simulink model "ofdm_txrx_supermimo_coded"  (Simulink 7.7)
settings: Solver=VariableStepDiscrete  SolverMode=SingleTasking  StartTime=0.0  StopTime=2*simOnly_simLength  RelTol=1e-3
PostLoadFcn:
  | ofdm_rx_supermimo_init
InitFcn:
  | ofdm_rx_supermimo_init
StartFcn:
  | ofdm_rx_supermimo_init
values from neighbouring files (symbols this model uses):
  ActionID_AFTransmit = 31    [ofdm_tx_supermimo_init.m]
  ActionID_Disabled = 0    [ofdm_tx_supermimo_init.m]
  ActionID_SetFlagA = 62    [ofdm_tx_supermimo_init.m]
  ActionID_SetFlagB = 61    [ofdm_tx_supermimo_init.m]
  BER_RAM_init_size = 2048    [ofdm_tx_supermimo_init.m]
  channel_h = [1 0; 1 0] * 0.3    [ofdm_tx_supermimo_init.m]
  CPLength = 16    [ofdm_tx_supermimo_init.m]
  longCorr_coef_i = [-4*real(LTS) 0]    [ofdm_rx_supermimo_init.m]
  longCorr_coef_q = [4*imag(LTS) 0]    [ofdm_rx_supermimo_init.m]
  max_CP_length = 16    [ofdm_tx_supermimo_init.m]
  max_num_baseRateSymbols = 31    [ofdm_tx_supermimo_init.m]
  max_num_subcarriers = 64    [ofdm_tx_supermimo_init.m]
  max_num_trainingSymbols = 15    [ofdm_tx_supermimo_init.m]
  max_numBytes = 16384    [ofdm_tx_supermimo_init.m]
  max_OFDM_symbols = 1023    [ofdm_tx_supermimo_init.m]
  modConstellation_qam16 = .75*[1 3 -1 -3]./3    [ofdm_tx_supermimo_init.m]
  modConstellation_qam64 = 0.875*[3 1 5 7 -3 -1 -5 -7]./7    [ofdm_tx_supermimo_init.m]
  modConstellation_qpsk = [1 -1]./sqrt(2)    [ofdm_tx_supermimo_init.m]
  numBaseRateSymbols = numBaseRateSymbols *2    [ofdm_tx_supermimo_init.m]
  numSubcarriers = 64    [ofdm_tx_supermimo_init.m]
  numTrainingSymbols = 2    [ofdm_tx_supermimo_init.m]
  pktTiming_count_payloadStart = pktTiming_count_lsStart + pktTiming_ls_num*pktTiming_ls_length    [ofdm_rx_supermimo_init.m]
  preamble = preamble_scale*[shortsyms_10 longsyms_2]    [ofdm_tx_supermimo_init.m]
  preamble_ROM = preamble_I_ROM + preamble_Q_ROM*2^16    [ofdm_tx_supermimo_init.m]
  RAM_init_size = max_numBytes    (= 16384)    [ofdm_tx_supermimo_init.m]
  RxDataScrambling_Seq = [40 198 78 63 82 173 102 245 48 111 172 115 147 230 216 93 72 65 62 2 205 242 122 90 128 83 105 97 73 10 5 252]    [ofdm_tx_supermimo_init.m]
  shortSymbol_time = shortSymbol_time(1:16).'    [ofdm_tx_supermimo_init.m]
  simOnly_numSamples = length(preamble)+( (numSubcarriers+CPLength)*(numTrainingSymbols + numBaseRateSymbols + numFullRateSymbols/2) )    [ofdm_tx_supermimo_init.m]
  train = [train train]    [ofdm_tx_supermimo_init.m]
  TxDataScrambling_Seq = [40 198 78 63 82 173 102 245 48 111 172 115 147 230 216 93 72 65 62 2 205 242 122 90 128 83 105 97 73 10 5 252]    [ofdm_tx_supermimo_init.m]
undefined symbols (not defined in the file or in its folder): AntA, AntB, BaseRate, Down, gcbh, numBuffs, numBytes, Up, xlBlockLoadCallback, xlGetNormalizedPeriod, xlipgetpartsetting
libraries: xbsIndex_r4 x5430
(part 23 of 49)

== system ofdm_txrx_supermimo_coded/OFDM Rx MIMO/Packet_Detection/RSSI-based PktDet/DIFS Counter ==
  Busy : Inport
  Idle : Inport  Port=2
  Convert1 : xbsIndex_r4/Convert  gui_display_data_type=Fixed-point  arith_type=Unsigned  n_bits=1  bin_pt=0  float_type=Single  exp_bits=8  fraction_bits=24  quantization=Truncate  overflow=Wrap  en=off  latency=0  dbl_ovrd=off  pipeline=off  block_type=convert
      settings: block_version=VER_STRING_GOES_HERE
  Convert2 : xbsIndex_r4/Convert  gui_display_data_type=Fixed-point  arith_type=Boolean  n_bits=1  bin_pt=0  float_type=Single  exp_bits=8  fraction_bits=24  quantization=Truncate  overflow=Wrap  en=off  latency=0  dbl_ovrd=off  pipeline=off  block_type=convert
      settings: block_version=VER_STRING_GOES_HERE
  Convert3 : xbsIndex_r4/Convert  gui_display_data_type=Fixed-point  arith_type=Boolean  n_bits=1  bin_pt=0  float_type=Single  exp_bits=8  fraction_bits=24  quantization=Truncate  overflow=Wrap  en=off  latency=0  dbl_ovrd=off  pipeline=off  block_type=convert
      settings: block_version=VER_STRING_GOES_HERE
  Counter1 : xbsIndex_r4/Counter  cnt_type=Free Running  cnt_to=Inf  operation=Up  start_count=0  cnt_by_val=1  arith_type=Unsigned  n_bits=10  bin_pt=0  load_pin=off  rst=on  en=on  explicit_period=off  period=4  dbl_ovrd=off  use_behavioral_HDL=off  implementation=Fabric  use_rpm=off  block_type=counter
  Inverter1 : xbsIndex_r4/Inverter  en=off  latency=0  dbl_ovrd=off  block_type=inv
      settings: xl_area=[0 0 0 0 0 0 0]  block_version=8.2
  Logical1 : xbsIndex_r4/Logical  logical_function=OR  inputs=2  en=off  latency=0  precision=Full  arith_type=Unsigned  n_bits=16  bin_pt=0  align_bp=on  dbl_ovrd=off  block_type=logical
  Relational3 : xbsIndex_r4/Relational  mode=a>b  en=off  latency=0  dbl_ovrd=off  block_type=relational
      settings: block_version=VER_STRING_GOES_HERE
  S-R Latch : Subsystem
  Idle for DIFS : Outport
  tag scope: regPktDet_idleCounter=global
  wires:
  Convert1/1 -> Idle for DIFS/1
  Idle/1 -> Convert2/1
  S-R Latch/1(Q) -> Convert1/1
  Relational3/1 -> S-R Latch/2(S)
  [regPktDet_DIFSperiod] -> Relational3/2
  Counter1/1 -> Relational3/1, [regPktDet_idleCounter]
  Inverter1/1 -> Logical1/2
  Logical1/1 -> Counter1/1
  Busy/1 -> Convert3/1
  Convert2/1 -> Counter1/2, Inverter1/1
  Convert3/1 -> S-R Latch/1(R), Logical1/1

== system ofdm_txrx_supermimo_coded/OFDM Rx MIMO/Packet_Detection/RSSI-based PktDet/MIMO Logic ==
  Det A : Inport
  Det B : Inport  Port=2
  Convert{1..4,7} (5 alike) : xbsIndex_r4/Convert  gui_display_data_type=Fixed-point  arith_type=Unsigned  n_bits=1  bin_pt=0  float_type=Single  exp_bits=8  fraction_bits=24  quantization=Truncate  overflow=Flag as error  en=off  latency=0  dbl_ovrd=off  pipeline=off  block_type=convert
      settings: block_version=10.1.3
  Logical : xbsIndex_r4/Logical  logical_function=AND  inputs=2  en=off  latency=0  precision=Full  arith_type=Unsigned  n_bits=16  bin_pt=0  align_bp=on  dbl_ovrd=off  block_type=logical
  Logical1 : xbsIndex_r4/Logical  logical_function=AND  inputs=2  en=off  latency=0  precision=Full  arith_type=Unsigned  n_bits=16  bin_pt=0  align_bp=on  dbl_ovrd=off  block_type=logical
  Logical2 : xbsIndex_r4/Logical  logical_function=OR  inputs=2  en=off  latency=0  precision=Full  arith_type=Unsigned  n_bits=16  bin_pt=0  align_bp=on  dbl_ovrd=off  block_type=logical
  Logical3 : xbsIndex_r4/Logical  logical_function=AND  inputs=2  en=off  latency=0  precision=Full  arith_type=Unsigned  n_bits=16  bin_pt=0  align_bp=on  dbl_ovrd=off  block_type=logical
  Mux : xbsIndex_r4/Mux  inputs=2  en=off  latency=0  precision=Full  arith_type=Unsigned  n_bits=16  bin_pt=14  quantization=Truncate  overflow=Wrap  dbl_ovrd=off  block_type=mux
  PktDet : Outport
  wires:
  [regPktDet_pktDetMode] -> Convert4/1
  [regPktDet_pktDetMask_A] -> Convert2/1
  [regPktDet_pktDetMask_B] -> Convert3/1
  Det A/1 -> Convert7/1
  Det B/1 -> Convert1/1
  Convert7/1 -> Logical/1
  Convert2/1 -> Logical/2
  Convert1/1 -> Logical1/1
  Convert3/1 -> Logical1/2
  Logical/1 -> Logical2/1, Logical3/1
  Logical1/1 -> Logical2/2, Logical3/2
  Logical2/1 -> Mux/3
  Convert4/1 -> Mux/1
  Logical3/1 -> Mux/2
  Mux/1 -> PktDet/1

== system ofdm_txrx_supermimo_coded/OFDM Rx MIMO/Packet_Detection/RSSI-based PktDet/Mid-Packet RSSI Register ==
  AntB : Inport
  AntA : Inport  Port=2
  Payload : Inport  Port=3
  Concat1 : xbsIndex_r4/Concat  num_inputs=2  dbl_ovrd=off  block_type=concat
      settings: block_version=10.1.3
  Convert4 : xbsIndex_r4/Convert  gui_display_data_type=Fixed-point  arith_type=Boolean  n_bits=1  bin_pt=0  float_type=Single  exp_bits=8  fraction_bits=24  quantization=Truncate  overflow=Wrap  en=off  latency=0  dbl_ovrd=off  pipeline=off  block_type=convert
      settings: block_version=10.1.3
  Convert5 : xbsIndex_r4/Convert  gui_display_data_type=Fixed-point  arith_type=Unsigned  n_bits=16  bin_pt=0  float_type=Single  exp_bits=8  fraction_bits=24  quantization=Truncate  overflow=Wrap  en=off  latency=0  dbl_ovrd=off  pipeline=off  block_type=convert
      settings: block_version=10.1.3
  Convert6 : xbsIndex_r4/Convert  gui_display_data_type=Fixed-point  arith_type=Unsigned  n_bits=16  bin_pt=0  float_type=Single  exp_bits=8  fraction_bits=24  quantization=Truncate  overflow=Wrap  en=off  latency=0  dbl_ovrd=off  pipeline=off  block_type=convert
      settings: block_version=10.1.3
  Terminator : Terminator
  To Register2 : xbsIndex_r4/To Register  shared_memory_name='midPacketRSSI'  init=0  ownership=Locally owned and initialized  explicit_data_type=on  gui_display_data_type=Fixed-point  arith_type=Unsigned  n_bits=32  bin_pt=0  preci_type=Single  dbl_ovrd=off  block_type=toreg
      settings: AttributesFormatString=<< %<shared_memory_name> >>  infoedit="Register block that writes data to a shared memory register.  Delay of one sample period."  block_version=10.1.3
  posedge1 : Subsystem
  wires:
  Payload/1 -> posedge1/1(In)
  posedge1/1(Out) -> Convert4/1
  Concat1/1 -> To Register2/1
  To Register2/1 -> Terminator/1
  Convert4/1 -> To Register2/2
  Convert5/1 -> Concat1/1
  Convert6/1 -> Concat1/2
  AntB/1 -> Convert5/1
  AntA/1 -> Convert6/1

== system ofdm_txrx_supermimo_coded/OFDM Rx MIMO/Packet_Detection/RSSI-based PktDet/RSSI Clock Generator ==
  note: The RSSI ADC on the radio board can be clocked up to 20MHz.
      | We clock it at 10MHz (1/4 the I/Q samping rate). This
      | clock is generated here by a 20MHz 2-bit counter. If the LBS is used,
      | the RSSI clock will be CLK/2 (10MHz). If the second LSB is used,
      | it will be CLK/4 (5MHz).
  2-bit Counter : xbsIndex_r4/Counter  cnt_type=Free Running  cnt_to=Inf  operation=Up  start_count=0  cnt_by_val=1  arith_type=Unsigned  n_bits=2  bin_pt=0  load_pin=off  rst=off  en=off  explicit_period=on  period=2  dbl_ovrd=off  use_behavioral_HDL=off  implementation=Fabric  use_rpm=off  block_type=counter
      settings: block_version=VER_STRING_GOES_HERE
  LSB : xbsIndex_r4/Slice  nbits=1  boolean_output=off  mode=Lower Bit Location + Width  bit1=0  base1=MSB of Input  bit0=0  base0=LSB of Input  dbl_ovrd=off  block_type=slice
      settings: infoedit="Extracts a given range of bits from each input sample and presents it at the output.  The output type is ordinarily unsigned with binary point at zero, but can be Boolean when the slice is one bit wide.<P><P>Hardware notes: In hardware this block costs nothing."  block_version=8.2.02
  LSB1 : xbsIndex_r4/Slice  nbits=1  boolean_output=off  mode=Lower Bit Location + Width  bit1=0  base1=MSB of Input  bit0=1  base0=LSB of Input  dbl_ovrd=off  block_type=slice
      settings: infoedit="Extracts a given range of bits from each input sample and presents it at the output.  The output type is ordinarily unsigned with binary point at zero, but can be Boolean when the slice is one bit wide.<P><P>Hardware notes: In hardware this block costs nothing."  block_version=8.2.02
  Mux : xbsIndex_r4/Mux  inputs=2  en=off  latency=0  precision=Full  arith_type=Unsigned  n_bits=16  bin_pt=14  quantization=Truncate  overflow=Wrap  dbl_ovrd=off  block_type=mux
      settings: block_version=8.2.02
  RSSI Clock : Scope
      settings: SaveName=ScopeData3
  RSSI_CLK_OUT : xbsIndex_r4/Gateway Out  inherit_from_input=off  hdl_port=on  timing_constraint=None  locs_specified=off  LOCs={}  UseAsDAC=off  DACChannel='1'  block_type=gatewayout
      settings: infoedit="Gateway out block.  Converts Xilinx fixed point inputs into ouputs of type Simulink integer, double, or fixed point.<P><P>Hardware notes:  In hardware these blocks become top level output ports or are discarded, depending on how they are configured."  block_version=VER_STRING_GOES_HERE
  wires:
  [regPktDet_RSSIclkRatioSel] -> Mux/1
  LSB1/1 -> Mux/3
  LSB/1 -> Mux/2
  Mux/1 -> RSSI_CLK_OUT/1
  RSSI_CLK_OUT/1 -> RSSI Clock/1
  2-bit Counter/1 -> LSB/1, LSB1/1

== system ofdm_txrx_supermimo_coded/OFDM Rx MIMO/Packet_Timing_Control/Disable for Interrupts/S-R Latch ==
  S : Inport
  R : Inport  Port=2
  Constant1 : xbsIndex_r4/Constant  const=1  gui_display_data_type=Fixed-point  arith_type=Boolean  n_bits=1  bin_pt=0  preci_type=Single  exp_width=8  frac_width=24  explicit_period=off  period=1  equ=P=C  opselect=C  inp2=PCIN>>17  opr=+  inp1=P  carry=CIN  dbl_ovrd=off  block_type=constant
      settings: block_version=VER_STRING_GOES_HERE
  Register2 : xbsIndex_r4/Register  init=0  rst=on  en=on  dbl_ovrd=off  block_type=register
      settings: xl_area=[1 1 0 0 0 0 0]  block_version=VER_STRING_GOES_HERE
  Q : Outport
  wires:
  Constant1/1 -> Register2/1
  R/1 -> Register2/2
  Register2/1 -> Q/1
  S/1 -> Register2/3

== system ofdm_txrx_supermimo_coded/OFDM Rx MIMO/Packet_Timing_Control/Ext Reset/debounce ==
  D : Inport
  Delay{,1..3} (4 alike) : xbsIndex_r4/Delay  rst=off  infoeditControl=Selection of Reset will increase slice count due to use of real FFs and instead of SRLs  en=off  latency=1  dbl_ovrd=off  reg_retiming=off  block_type=delay
      settings: infoedit=Hardware notes: A delay line is a chain, each link of which is an SRL16 followed by a flip-flop. If register retiming is enabled, the delay line is a chain of flip-flops.  xl_area=[1 1 0 0 0 0 0]  block_version=8.2
  Logical : xbsIndex_r4/Logical  logical_function=AND  inputs=5  en=off  latency=0  precision=Full  arith_type=Unsigned  n_bits=16  bin_pt=0  align_bp=on  dbl_ovrd=off  block_type=logical
      settings: xl_area=[1 0 0 1 0 0 0]  block_version=8.2
  Q : Outport
  wires:
  Logical/1 -> Q/1
  D/1 -> Delay/1, Logical/1
  Delay/1 -> Delay1/1, Logical/2
  Delay1/1 -> Delay2/1, Logical/3
  Delay2/1 -> Delay3/1, Logical/4
  Delay3/1 -> Logical/5

== system ofdm_txrx_supermimo_coded/OFDM Tx MIMO/OutputBuffers/Addr Gen/1 Shift Reg ==
  d : Inport
  rst : Inport  Port=2
  en : Inport  Port=3
  Payload Running1 : xbsIndex_r4/Register  init=0  rst=on  en=on  dbl_ovrd=off  block_type=register
      settings: xl_area=[1 1 0 0 0 0 0]  block_version=VER_STRING_GOES_HERE
  Q : Outport
  wires:
  en/1 -> Payload Running1/3
  rst/1 -> Payload Running1/2
  d/1 -> Payload Running1/1
  Payload Running1/1 -> Q/1

== system ofdm_txrx_supermimo_coded/OFDM Tx MIMO/OutputBuffers/Addr Gen/1 Shift Reg1 ==
  d : Inport
  rst : Inport  Port=2
  en : Inport  Port=3
  Payload Running1 : xbsIndex_r4/Register  init=1  rst=on  en=on  dbl_ovrd=off  block_type=register
      settings: xl_area=[1 1 0 0 0 0 0]  block_version=VER_STRING_GOES_HERE
  Q : Outport
  wires:
  Payload Running1/1 -> Q/1
  d/1 -> Payload Running1/1
  rst/1 -> Payload Running1/2
  en/1 -> Payload Running1/3

== system ofdm_txrx_supermimo_coded/OFDM Tx MIMO/OutputBuffers/Addr Gen/2 Shift Reg ==
  d : Inport
  en : Inport  Port=2
  rst : Inport  Port=3
  Payload Running1 : xbsIndex_r4/Register  init=0  rst=on  en=on  dbl_ovrd=off  block_type=register
      settings: xl_area=[1 1 0 0 0 0 0]  block_version=VER_STRING_GOES_HERE
  Payload Running3 : xbsIndex_r4/Register  init=0  rst=on  en=on  dbl_ovrd=off  block_type=register
      settings: xl_area=[1 1 0 0 0 0 0]  block_version=VER_STRING_GOES_HERE
  Q : Outport
  wires:
  en/1 -> Payload Running1/3, Payload Running3/3
  rst/1 -> Payload Running1/2, Payload Running3/2
  d/1 -> Payload Running1/1
  Payload Running3/1 -> Q/1
  Payload Running1/1 -> Payload Running3/1

== system ofdm_txrx_supermimo_coded/OFDM Tx MIMO/OutputBuffers/Addr Gen/Oscillator1 ==
  en : Inport
  rst : Inport  Port=2
  Assert : xbsIndex_r4/Assert  assert_type=on  type_source=Explicitly  gui_display_data_type=Fixed-point  arith_type=Boolean  n_bits=16  bin_pt=14  preci_type=Single  exp_width=8  frac_width=24  assert_rate=on  rate_source=Explicitly  period=2  output_port=on  block_type=assert
      settings: infoedit=Asserts a user-defined sample rate and/or type on a signal.<P><P>Hardware notes: In hardware this block costs nothing.  block_version=VER_STRING_GOES_HERE
  Inverter1 : xbsIndex_r4/Inverter  en=off  latency=0  dbl_ovrd=off  block_type=inv
      settings: block_version=VER_STRING_GOES_HERE
  Register : xbsIndex_r4/Register  init=0  rst=on  en=on  dbl_ovrd=off  block_type=register
      settings: block_version=VER_STRING_GOES_HERE
  Q : Outport
  wires:
  Register/1 -> Assert/1, Q/1
  en/1 -> Register/3
  Inverter1/1 -> Register/1
  Assert/1 -> Inverter1/1
  rst/1 -> Register/2

== system ofdm_txrx_supermimo_coded/OFDM Tx MIMO/OutputBuffers/Addr Gen/Read Addr Generator ==
  note: About the addresses:
      | The IFFT output time series [0,1,...63] is written to a RAM with an offset of 16, modulo 64, so that:
      | Xn=[0,1,...63] -> WrAddr=[16,17,...15]
      |
      | This allows much easier read address generation, which must include both the full IFFT output and a cyclic prefix.
      |
      | For SISO/Multiplexing MIMO modes, the output series per symbol must be:
      | Xn_out = [48,49...63,0,1,...63] - 80 samples long ({CP SYM} = {SYM[48:63] SYM[0:63]})
      |
      | Thus, the 80-sample sequence of read addresses is
      | RdAddr=[0,1,...15,16,17,...63,0,1,...15]
      |
      | For Alamouti mode, the non-conjugated symbols are handled the same as the SISO/Multiplexing mode. For the
      | conjugated symbols, we use the Fourier identity of:
      | if x(t) = ifft(X(f)) then
      | ifft(conj(X(f))) = conj(x(-t))
      |
      | So we can "generate" the conjugate of the frequency domain symbol by reading the time domain series (which we
      | already have, since Alamouti uses the same symbol twice) backwards, then conjugating. Notice that the zero'th entry
      | in the time series doesn't change, but the rest do.
      |
      | So, for conjugated Alamouti symbols:
      | Xn_out = [16,15,14,...0,63,...2,1] - 80 saples long {CP SYM} = {SYM[16:1] SYM[0] SYM[63:1]}
      | Thus,
      | RdAddr = [32,31,...0,63,62,...17]
  En : Inport
  Rst : Inport  Port=2
  SwapDir : Inport  Port=3
  6LSB : xbsIndex_r4/Slice  nbits=log2(numSubcarriers)  boolean_output=off  mode=Lower Bit Location + Width  bit1=0  base1=MSB of Input  bit0=0  base0=LSB of Input  dbl_ovrd=off  block_type=slice
      settings: infoedit="Extracts a given range of bits from each input sample and presents it at the output.  The output type is ordinarily unsigned with binary point at zero, but can be Boolean when the slice is one bit wide.<P><P>Hardware notes: In hardware this block costs nothing."  block_version=VER_STRING_GOES_HERE
  6LSB1 : xbsIndex_r4/Slice  nbits=log2(numSubcarriers)  boolean_output=off  mode=Lower Bit Location + Width  bit1=0  base1=MSB of Input  bit0=0  base0=LSB of Input  dbl_ovrd=off  block_type=slice
      settings: infoedit="Extracts a given range of bits from each input sample and presents it at the output.  The output type is ordinarily unsigned with binary point at zero, but can be Boolean when the slice is one bit wide.<P><P>Hardware notes: In hardware this block costs nothing."  block_version=VER_STRING_GOES_HERE
  AddSub : xbsIndex_r4/AddSub  mode=Addition  use_carryin=off  use_carryout=off  en=off  latency=0  precision=Full  arith_type=Unsigned  n_bits=16  bin_pt=14  quantization=Truncate  overflow=Wrap  dbl_ovrd=off  use_behavioral_HDL=off  hw_selection=Fabric  pipelined=off  use_rpm=on  block_type=addsub
      settings: block_version=8.2.02
  Constant1 : xbsIndex_r4/Constant  const=32  gui_display_data_type=Fixed-point  arith_type=Unsigned  n_bits=ceil(log2(numSubcarriers + CPLength - 1))  bin_pt=0  preci_type=Single  exp_width=8  frac_width=24  explicit_period=off  period=2  equ=P=C  opselect=C  inp2=PCIN>>17  opr=+  inp1=P  carry=CIN  dbl_ovrd=off  block_type=constant
      settings: block_version=VER_STRING_GOES_HERE
  Constant3 : xbsIndex_r4/Constant  const=numSubcarriers + CPLength - 1  gui_display_data_type=Fixed-point  arith_type=Unsigned  n_bits=ceil(log2(numSubcarriers + CPLength - 1))  bin_pt=0  preci_type=Single  exp_width=8  frac_width=24  explicit_period=off  period=2  equ=P=C  opselect=C  inp2=PCIN>>17  opr=+  inp1=P  carry=CIN  dbl_ovrd=off  block_type=constant
      settings: block_version=VER_STRING_GOES_HERE
  Down Sample2 : xbsIndex_r4/Down Sample  sample_ratio=2  sample_phase=First Value of Frame  en=off  latency=0  dbl_ovrd=off  block_type=dsamp
      settings: block_version=VER_STRING_GOES_HERE
  Logical : xbsIndex_r4/Logical  logical_function=OR  inputs=2  en=off  latency=0  precision=Full  arith_type=Unsigned  n_bits=16  bin_pt=0  align_bp=on  dbl_ovrd=off  block_type=logical
      settings: block_version=VER_STRING_GOES_HERE
  Mux : xbsIndex_r4/Mux  inputs=2  en=off  latency=0  precision=Full  arith_type=Unsigned  n_bits=16  bin_pt=14  quantization=Truncate  overflow=Wrap  dbl_ovrd=off  block_type=mux
      settings: block_version=8.2.02
  Negate : xbsIndex_r4/Negate  precision=Full  arith_type="Signed  (2's comp)"  n_bits=16  bin_pt=14  quantization=Truncate  overflow=Wrap  en=off  latency=0  dbl_ovrd=off  block_type=negate
      settings: block_version=8.2.02
  Read Counter : xbsIndex_r4/Counter  cnt_type=Free Running  cnt_to=numSubcarriers + CPLength - 1  operation=Up  start_count=0  cnt_by_val=1  arith_type=Unsigned  n_bits=ceil(log2(numSubcarriers + CPLength - 1))  bin_pt=0  load_pin=off  rst=on  en=on  explicit_period=off  period=2  dbl_ovrd=off  use_behavioral_HDL=off  implementation=Fabric  use_rpm=off  block_type=counter
      settings: block_version=VER_STRING_GOES_HERE
  Relational : xbsIndex_r4/Relational  mode=a=b  en=off  latency=0  dbl_ovrd=off  block_type=relational
      settings: block_version=VER_STRING_GOES_HERE
  Up Sample : xbsIndex_r4/Up Sample  sample_ratio=2  copy_samples=off  en=off  latency=0  dbl_ovrd=off  block_type=usamp
      settings: infoedit="Up samples input data.  Inserted values  can be zeros or copies of the most recent input sample.<P><P>Hardware notes: No hardware is needed if inserted values are copies of the input sample; otherwise, a mux and single bit flip-flop are used."  block_version=VER_STRING_GOES_HERE
  Q : Outport
  Ovfl : Outport  Port=2
  Slot : Outport  Port=3
  wires:
  Read Counter/1 -> Relational/1, AddSub/1, 6LSB/1
  Relational/1 -> Logical/2, Up Sample/1
  Logical/1 -> Read Counter/1
  Constant3/1 -> Relational/2
  En/1 -> Read Counter/2
  Rst/1 -> Logical/1
  Up Sample/1 -> Ovfl/1
  AddSub/1 -> Negate/1
  Negate/1 -> 6LSB1/1
  Constant1/1 -> AddSub/2
  Mux/1 -> Q/1
  6LSB/1 -> Mux/2
  6LSB1/1 -> Mux/3
  SwapDir/1 -> Down Sample2/1
  Down Sample2/1 -> Mux/1, Slot/1

== system ofdm_txrx_supermimo_coded/OFDM Tx MIMO/OutputBuffers/Addr Gen/SR Latch ==
  S : Inport
  R : Inport  Port=2
  Constant1 : xbsIndex_r4/Constant  const=1  gui_display_data_type=Fixed-point  arith_type=Boolean  n_bits=1  bin_pt=0  preci_type=Single  exp_width=8  frac_width=24  explicit_period=off  period=2  equ=P=C  opselect=C  inp2=PCIN>>17  opr=+  inp1=P  carry=CIN  dbl_ovrd=off  block_type=constant
      settings: block_version=VER_STRING_GOES_HERE
  Payload Running3 : xbsIndex_r4/Register  init=0  rst=on  en=on  dbl_ovrd=off  block_type=register
      settings: xl_area=[1 1 0 0 0 0 0]  block_version=VER_STRING_GOES_HERE
  Q : Outport
  wires:
  Constant1/1 -> Payload Running3/1
  R/1 -> Payload Running3/2
  S/1 -> Payload Running3/3
  Payload Running3/1 -> Q/1

== system ofdm_txrx_supermimo_coded/OFDM Tx MIMO/OutputBuffers/Addr Gen/Subsystem ==
  note: Alamouti mode treats base rate and full rate symbols
      | the same, so the memory address generation logic
      | ignore BaseRate for Alamouti transmissions.
  BaseRate : Inport
  Rst : Inport  Port=2
  En : Inport  Port=3
  Training : Inport  Port=4
  Mux : xbsIndex_r4/Mux  inputs=2  en=off  latency=0  precision=Full  arith_type=Unsigned  n_bits=16  bin_pt=14  quantization=Truncate  overflow=Wrap  dbl_ovrd=off  block_type=mux
  Oscillator : Subsystem
  Oscillator2 : Subsystem
  Q : Outport
  wires:
  Oscillator2/1(Q) -> Mux/2  "Wr Bank Sel"
  Oscillator/1(Q) -> Mux/3
  BaseRate/1 -> Oscillator2/1(period)
  Rst/1 -> Oscillator2/2(rst), Oscillator/1(rst)
  En/1 -> Oscillator2/3(en), Oscillator/2(en)
  Training/1 -> Oscillator/3(Period)
  Mux/1 -> Q/1
  [TxReg_Alamouti_Mode] -> Mux/1

== system ofdm_txrx_supermimo_coded/OFDM Tx MIMO/OutputBuffers/RAMs/Alamouti Encoding ==
  note: In Alamouti mode, even and odd syms are sent via
      | both antennas, in anternating order. In MIMO mode,
      | even/odd syms are mapped to antennas A/B.
  Slot : Inport
  EvenSyms_I : Inport  Port=2
  EvenSyms_Q : Inport  Port=3
  OddSyms_I : Inport  Port=4
  OddSyms_Q : Inport  Port=5
  Mux{,1..3} (4 alike) : xbsIndex_r4/Mux  inputs=2  en=off  latency=0  precision=Full  arith_type=Unsigned  n_bits=16  bin_pt=14  quantization=Truncate  overflow=Wrap  dbl_ovrd=off  block_type=mux
      settings: block_version=8.2.02
  Negate : xbsIndex_r4/Negate  precision=User Defined  arith_type="Signed  (2's comp)"  n_bits=14  bin_pt=13  quantization=Truncate  overflow=Saturate  en=off  latency=0  dbl_ovrd=off  block_type=negate
      settings: block_version=8.2.02
  Negate1 : xbsIndex_r4/Negate  precision=User Defined  arith_type="Signed  (2's comp)"  n_bits=14  bin_pt=13  quantization=Truncate  overflow=Saturate  en=off  latency=0  dbl_ovrd=off  block_type=negate
      settings: block_version=8.2.02
  AntA_I : Outport
  AntA_Q : Outport  Port=2
  AntB_I : Outport  Port=3
  AntB_Q : Outport  Port=4
  wires:
  Mux/1 -> AntB_Q/1
  Mux3/1 -> AntA_I/1
  Mux2/1 -> AntA_Q/1
  Mux1/1 -> AntB_I/1
  Slot/1 -> Mux1/1, Mux/1, Mux2/1, Mux3/1
  EvenSyms_I/1 -> Mux3/2, Mux1/3
  EvenSyms_Q/1 -> Mux2/2, Negate1/1
  OddSyms_I/1 -> Mux1/2, Negate/1
  OddSyms_Q/1 -> Mux/2, Mux2/3
  Negate/1 -> Mux3/3
  Negate1/1 -> Mux/3

== system ofdm_txrx_supermimo_coded/OFDM Tx MIMO/OutputBuffers/RAMs/Even Syms RAM ==
  I : Inport
  Q : Inport  Port=2
  We : Inport  Port=3
  Wr Addr : Inport  Port=4
  Rd Addr : Inport  Port=5
  OE# : Inport  Port=6
  Constant8 : xbsIndex_r4/Constant  const=0  gui_display_data_type=Fixed-point  arith_type=Boolean  n_bits=1  bin_pt=0  preci_type=Single  exp_width=8  frac_width=24  explicit_period=off  period=1  equ=P=C  opselect=C  inp2=PCIN>>17  opr=+  inp1=P  carry=CIN  dbl_ovrd=off  block_type=constant
      settings: block_version=VER_STRING_GOES_HERE
  Dual Port RAM : xbsIndex_r4/Dual Port RAM  depth=2*numSubcarriers  initVector=[repmat(0.25,1,64) repmat(0.5,1,64)]  distributed_mem=Block RAM  init_a=0  init_b=0  rst_a=off  rst_b=on  en_a=off  en_b=off  latency=1  write_mode_A=Read Before Write  write_mode_B=Read Before Write  dbl_ovrd=off  optimize=Area  use_rpm=off  block_type=dpram
      settings: xl_area=[0 0 1 0 0 0 0]  block_version=VER_STRING_GOES_HERE
  Dual Port RAM1 : xbsIndex_r4/Dual Port RAM  depth=2*numSubcarriers  initVector=[repmat(0.25,1,64) repmat(0.5,1,64)]  distributed_mem=Block RAM  init_a=0  init_b=0  rst_a=off  rst_b=on  en_a=off  en_b=off  latency=1  write_mode_A=Read Before Write  write_mode_B=Read Before Write  dbl_ovrd=off  optimize=Area  use_rpm=off  block_type=dpram
      settings: xl_area=[0 0 1 0 0 0 0]  block_version=VER_STRING_GOES_HERE
  " I" : Outport
  " Q" : Outport  Port=2
  wires:
  OE#/1 -> Dual Port RAM1/7, Dual Port RAM/7
  We/1 -> Dual Port RAM1/3, Dual Port RAM/3
  Q/1 -> Dual Port RAM1/2, Dual Port RAM1/5
  I/1 -> Dual Port RAM/2, Dual Port RAM/5
  Dual Port RAM/2 -> " I"/1
  Dual Port RAM1/2 -> " Q"/1
  Rd Addr/1 -> Dual Port RAM/4, Dual Port RAM1/4
  Constant8/1 -> Dual Port RAM1/6, Dual Port RAM/6
  Wr Addr/1 -> Dual Port RAM/1, Dual Port RAM1/1

== system ofdm_txrx_supermimo_coded/OFDM Tx MIMO/OutputBuffers/RAMs/Odd Syms RAM ==
  I : Inport
  Q : Inport  Port=2
  We : Inport  Port=3
  Wr Addr : Inport  Port=4
  Rd Addr : Inport  Port=5
  OE# : Inport  Port=6
  Constant8 : xbsIndex_r4/Constant  const=0  gui_display_data_type=Fixed-point  arith_type=Boolean  n_bits=1  bin_pt=0  preci_type=Single  exp_width=8  frac_width=24  explicit_period=off  period=1  equ=P=C  opselect=C  inp2=PCIN>>17  opr=+  inp1=P  carry=CIN  dbl_ovrd=off  block_type=constant
      settings: block_version=VER_STRING_GOES_HERE
  Dual Port RAM : xbsIndex_r4/Dual Port RAM  depth=2*numSubcarriers  initVector=-[repmat(0.25,1,64) repmat(0.5,1,64)]  distributed_mem=Block RAM  init_a=0  init_b=0  rst_a=off  rst_b=on  en_a=off  en_b=off  latency=1  write_mode_A=Read Before Write  write_mode_B=Read Before Write  dbl_ovrd=off  optimize=Area  use_rpm=off  block_type=dpram
      settings: xl_area=[0 0 1 0 0 0 0]  block_version=VER_STRING_GOES_HERE
  Dual Port RAM1 : xbsIndex_r4/Dual Port RAM  depth=2*numSubcarriers  initVector=-[repmat(0.25,1,64) repmat(0.5,1,64)]  distributed_mem=Block RAM  init_a=0  init_b=0  rst_a=off  rst_b=on  en_a=off  en_b=off  latency=1  write_mode_A=Read Before Write  write_mode_B=Read Before Write  dbl_ovrd=off  optimize=Area  use_rpm=off  block_type=dpram
      settings: xl_area=[0 0 1 0 0 0 0]  block_version=VER_STRING_GOES_HERE
  " I" : Outport
  " Q" : Outport  Port=2
  wires:
  Wr Addr/1 -> Dual Port RAM1/1, Dual Port RAM/1
  Constant8/1 -> Dual Port RAM/6, Dual Port RAM1/6
  Rd Addr/1 -> Dual Port RAM1/4, Dual Port RAM/4
  Dual Port RAM1/2 -> " Q"/1
  Dual Port RAM/2 -> " I"/1
  I/1 -> Dual Port RAM/5, Dual Port RAM/2
  Q/1 -> Dual Port RAM1/5, Dual Port RAM1/2
  We/1 -> Dual Port RAM1/3, Dual Port RAM/3
  OE#/1 -> Dual Port RAM/7, Dual Port RAM1/7

== system ofdm_txrx_supermimo_coded/OFDM Tx MIMO/OutputMuxes/Antenna Swapping/PreambleEn ==
  R : Inport
  S : Inport  Port=2
  Constant4 : xbsIndex_r4/Constant  const=1  gui_display_data_type=Fixed-point  arith_type=Unsigned  n_bits=1  bin_pt=0  preci_type=Single  exp_width=8  frac_width=24  explicit_period=off  period=2  equ=P=C  opselect=C  inp2=PCIN>>17  opr=+  inp1=P  carry=CIN  dbl_ovrd=off  block_type=constant
      settings: block_version=VER_STRING_GOES_HERE
  Convert : xbsIndex_r4/Convert  gui_display_data_type=Fixed-point  arith_type=Boolean  n_bits=16  bin_pt=14  float_type=Single  exp_bits=8  fraction_bits=24  quantization=Truncate  overflow=Wrap  en=off  latency=0  dbl_ovrd=off  pipeline=off  block_type=convert
      settings: block_version=10.1.3
  Convert1 : xbsIndex_r4/Convert  gui_display_data_type=Fixed-point  arith_type=Boolean  n_bits=16  bin_pt=14  float_type=Single  exp_bits=8  fraction_bits=24  quantization=Truncate  overflow=Wrap  en=off  latency=0  dbl_ovrd=off  pipeline=off  block_type=convert
      settings: block_version=10.1.3
  Register1 : xbsIndex_r4/Register  init=0  rst=on  en=on  dbl_ovrd=off  block_type=register
      settings: xl_area=[5 10 0 0 0 0 0]  block_version=VER_STRING_GOES_HERE
  Q : Outport
  wires:
  Convert1/1 -> Register1/3
  Convert/1 -> Register1/2
  S/1 -> Convert1/1
  Register1/1 -> Q/1
  R/1 -> Convert/1
  Constant4/1 -> Register1/1

== system ofdm_txrx_supermimo_coded/OFDM Tx MIMO/OutputMuxes/Antenna Swapping/posedge3 ==
  D : Inport
  Delay : xbsIndex_r4/Delay  rst=off  infoeditControl=Selection of Reset will increase slice count due to use of real FFs and instead of SRLs  en=off  latency=1  dbl_ovrd=off  reg_retiming=off  block_type=delay
      settings: infoedit=Hardware notes: A delay line is a chain, each link of which is an SRL16 followed by a flip-flop. If register retiming is enabled, the delay line is a chain of flip-flops.  xl_area=[1 1 0 0 0 0 0]  block_version=VER_STRING_GOES_HERE
  Inverter : xbsIndex_r4/Inverter  en=off  latency=0  dbl_ovrd=off  block_type=inv
      settings: xl_area=[0 0 0 0 0 0 0]  block_version=VER_STRING_GOES_HERE
  Logical1 : xbsIndex_r4/Logical  logical_function=AND  inputs=2  en=off  latency=0  precision=Full  arith_type=Unsigned  n_bits=8  bin_pt=2  align_bp=on  dbl_ovrd=off  block_type=logical
      settings: xl_area=[1 0 0 1 0 0 0]  block_version=VER_STRING_GOES_HERE
  Q : Outport
  wires:
  Logical1/1 -> Q/1
  Delay/1 -> Inverter/1
  D/1 -> Delay/1, Logical1/2
  Inverter/1 -> Logical1/1

== system ofdm_txrx_supermimo_coded/OFDM Tx MIMO/OutputMuxes/Preamble Generation/Addr Gen ==
  note: Add either 8 or 24 to the sample index
      | to jump past the cyclic padding in
      | the preamble buffer
  note: Add another offset for antenna B
  Samp Ind : Inport
  AddSub3 : xbsIndex_r4/AddSub  mode=Addition  use_carryin=off  use_carryout=off  en=off  latency=0  precision=User Defined  arith_type=Unsigned  n_bits=9  bin_pt=0  quantization=Truncate  overflow=Wrap  dbl_ovrd=off  use_behavioral_HDL=off  hw_selection=Fabric  pipelined=off  use_rpm=on  block_type=addsub
      settings: block_version=8.2.02
  AddSub5 : xbsIndex_r4/AddSub  mode=Addition  use_carryin=off  use_carryout=off  en=off  latency=0  precision=User Defined  arith_type=Unsigned  n_bits=9  bin_pt=0  quantization=Truncate  overflow=Wrap  dbl_ovrd=off  use_behavioral_HDL=off  hw_selection=Fabric  pipelined=off  use_rpm=on  block_type=addsub
      settings: block_version=8.2.02
  Concat : xbsIndex_r4/Concat  num_inputs=3  dbl_ovrd=off  block_type=concat
      settings: block_version=10.1.3
  Constant1 : xbsIndex_r4/Constant  const=1  gui_display_data_type=Fixed-point  arith_type=Unsigned  n_bits=1  bin_pt=0  preci_type=Single  exp_width=8  frac_width=24  explicit_period=off  period=1  equ=P=C  opselect=C  inp2=PCIN>>17  opr=+  inp1=P  carry=CIN  dbl_ovrd=off  block_type=constant
  Constant4 : xbsIndex_r4/Constant  const=0  gui_display_data_type=Fixed-point  arith_type=Unsigned  n_bits=3  bin_pt=0  preci_type=Single  exp_width=8  frac_width=24  explicit_period=off  period=1  equ=P=C  opselect=C  inp2=PCIN>>17  opr=+  inp1=P  carry=CIN  dbl_ovrd=off  block_type=constant
  Constant5 : xbsIndex_r4/Constant  const=(10 * length(shortSymbol_time))  gui_display_data_type=Fixed-point  arith_type=Unsigned  n_bits=8  bin_pt=0  preci_type=Single  exp_width=8  frac_width=24  explicit_period=off  period=1  equ=P=C  opselect=C  inp2=PCIN>>17  opr=+  inp1=P  carry=CIN  dbl_ovrd=off  block_type=constant
  Down Sample : xbsIndex_r4/Down Sample  sample_ratio=2  sample_phase="Last Value of Frame  (most efficient)"  en=off  latency=1  dbl_ovrd=off  block_type=dsamp
      settings: block_version=VER_STRING_GOES_HERE
  Relational1 : xbsIndex_r4/Relational  mode=a>=b  en=off  latency=0  dbl_ovrd=off  block_type=relational
      settings: block_version=10.1.3
  AddrA : Outport
  AddrB : Outport  Port=2
  LTS : Outport  Port=3
  wires:
  [TxReg_AntBPreambleShift] -> Down Sample/1
  Samp Ind/1 -> Relational1/1, AddSub5/1
  Constant5/1 -> Relational1/2
  Relational1/1 -> Concat/1, LTS/1
  Concat/1 -> AddSub5/2
  AddSub5/1 -> AddrA/1, AddSub3/1
  Down Sample/1 -> AddSub3/2
  AddSub3/1 -> AddrB/1
  Constant4/1 -> Concat/3
  Constant1/1 -> Concat/2

== system ofdm_txrx_supermimo_coded/OFDM Tx MIMO/OutputMuxes/TxDone Logic/Copy_Downsample ==
  D : Inport
  Delay : xbsIndex_r4/Delay  rst=off  infoeditControl=Selection of Reset will increase slice count due to use of real FFs and instead of SRLs  en=off  latency=1  dbl_ovrd=off  reg_retiming=off  block_type=delay
      settings: infoedit=Hardware notes: A delay line is a chain, each link of which is an SRL16 followed by a flip-flop. If register retiming is enabled, the delay line is a chain of flip-flops.  block_version=VER_STRING_GOES_HERE
  Down Sample : xbsIndex_r4/Down Sample  sample_ratio=2  sample_phase="Last Value of Frame  (most efficient)"  en=off  latency=1  dbl_ovrd=off  block_type=dsamp
      settings: block_version=VER_STRING_GOES_HERE
  Logical : xbsIndex_r4/Logical  logical_function=OR  inputs=2  en=off  latency=0  precision=Full  arith_type=Unsigned  n_bits=16  bin_pt=0  align_bp=on  dbl_ovrd=off  block_type=logical
      settings: block_version=VER_STRING_GOES_HERE
  Q : Outport
  wires:
  Down Sample/1 -> Q/1
  Logical/1 -> Down Sample/1
  D/1 -> Logical/2, Delay/1
  Delay/1 -> Logical/1

== system ofdm_txrx_supermimo_coded/OFDM Tx MIMO/OutputMuxes/TxRunning Outputs/Delay ==
  note: Delay stretches output pulse to a few cycles.
  Tx : Inport
  Rst : Inport  Port=2
  Counter : xbsIndex_r4/Counter  cnt_type=Free Running  cnt_to=Inf  operation=Up  start_count=0  cnt_by_val=1  arith_type=Unsigned  n_bits=9  bin_pt=0  load_pin=off  rst=on  en=on  explicit_period=off  period=1  dbl_ovrd=off  use_behavioral_HDL=off  implementation=Fabric  use_rpm=off  block_type=counter
  Delay1 : xbsIndex_r4/Delay  rst=off  infoeditControl=Selection of Reset will increase slice count due to use of real FFs and instead of SRLs  en=off  latency=2  dbl_ovrd=off  reg_retiming=off  block_type=delay
      settings: infoedit=Hardware notes: A delay line is a chain, each link of which is an SRL16 followed by a flip-flop. If register retiming is enabled, the delay line is a chain of flip-flops.  block_version=VER_STRING_GOES_HERE
  Inverter : xbsIndex_r4/Inverter  en=off  latency=0  dbl_ovrd=off  block_type=inv
      settings: xl_area=[0 0 0 0 0 0 0]  block_version=8.2
  Logical4 : xbsIndex_r4/Logical  logical_function=OR  inputs=2  en=off  latency=0  precision=Full  arith_type=Unsigned  n_bits=16  bin_pt=0  align_bp=on  dbl_ovrd=off  block_type=logical
  Relational : xbsIndex_r4/Relational  mode=a>b  en=off  latency=0  dbl_ovrd=off  block_type=relational
      settings: block_version=VER_STRING_GOES_HERE
  S-R Latch4 : Subsystem
  Tx_d : Outport
  dly : Outport  Port=2
  wires:
  S-R Latch4/1(Q) -> Counter/2, Inverter/1
  Counter/1 -> Relational/1
  Inverter/1 -> Counter/1
  Tx/1 -> S-R Latch4/1(S)
  Relational/1 -> Tx_d/1, Delay1/1
  Logical4/1 -> S-R Latch4/2(R)
  Rst/1 -> Logical4/1
  [TxReg_TxRunningOut_delay] -> Relational/2, dly/1
  Delay1/1 -> Logical4/2

== system ofdm_txrx_supermimo_coded/OFDM Tx MIMO/OutputMuxes/TxRunning Outputs/posedge2 ==
  In : Inport
  Delay : xbsIndex_r4/Delay  rst=off  infoeditControl=Selection of Reset will increase slice count due to use of real FFs and instead of SRLs  en=off  latency=1  dbl_ovrd=off  reg_retiming=off  block_type=delay
      settings: infoedit=Hardware notes: A delay line is a chain, each link of which is an SRL16 followed by a flip-flop. If register retiming is enabled, the delay line is a chain of flip-flops.  xl_area=[1 1 0 0 0 0 0]  block_version=8.2
  Inverter : xbsIndex_r4/Inverter  en=off  latency=0  dbl_ovrd=off  block_type=inv
      settings: xl_area=[0 0 0 0 0 0 0]  block_version=8.2
  Logical : xbsIndex_r4/Logical  logical_function=AND  inputs=2  en=off  latency=0  precision=Full  arith_type=Unsigned  n_bits=16  bin_pt=0  align_bp=on  dbl_ovrd=off  block_type=logical
      settings: xl_area=[1 0 0 1 0 0 0]  block_version=8.2
  Out : Outport
  wires:
  Delay/1 -> Inverter/1
  Inverter/1 -> Logical/2
  In/1 -> Logical/1, Delay/1
  Logical/1 -> Out/1

== system ofdm_txrx_supermimo_coded/OFDM Tx MIMO/Training_Data/Ant Selector/Oscillator ==
  rst : Inport
  en : Inport  Port=2
  Assert : xbsIndex_r4/Assert  assert_type=on  type_source=Explicitly  gui_display_data_type=Fixed-point  arith_type=Boolean  n_bits=16  bin_pt=14  preci_type=Single  exp_width=8  frac_width=24  assert_rate=on  rate_source=Explicitly  period=2  output_port=on  block_type=assert
      settings: infoedit=Asserts a user-defined sample rate and/or type on a signal.<P><P>Hardware notes: In hardware this block costs nothing.  block_version=VER_STRING_GOES_HERE
  Inverter1 : xbsIndex_r4/Inverter  en=off  latency=0  dbl_ovrd=off  block_type=inv
      settings: block_version=VER_STRING_GOES_HERE
  Register : xbsIndex_r4/Register  init=0  rst=on  en=on  dbl_ovrd=off  block_type=register
      settings: block_version=VER_STRING_GOES_HERE
  Q : Outport
  wires:
  en/1 -> Register/3
  Inverter1/1 -> Register/1
  Assert/1 -> Inverter1/1
  Register/1 -> Assert/1, Q/1
  rst/1 -> Register/2

== system ofdm_txrx_supermimo_coded/OFDM Tx MIMO/Training_Data/Ant Selector/negedge ==
  D : Inport
  Delay : xbsIndex_r4/Delay  rst=off  infoeditControl=Selection of Reset will increase slice count due to use of real FFs and instead of SRLs  en=off  latency=1  dbl_ovrd=off  reg_retiming=on  block_type=delay
      settings: infoedit=Hardware notes: A delay line is a chain, each link of which is an SRL16 followed by a flip-flop. If register retiming is enabled, the delay line is a chain of flip-flops.  block_version=VER_STRING_GOES_HERE
  Inverter1 : xbsIndex_r4/Inverter  en=off  latency=0  dbl_ovrd=off  block_type=inv
      settings: block_version=VER_STRING_GOES_HERE
  Logical3 : xbsIndex_r4/Logical  logical_function=AND  inputs=2  en=off  latency=0  precision=Full  arith_type=Unsigned  n_bits=16  bin_pt=0  align_bp=on  dbl_ovrd=off  block_type=logical
      settings: block_version=VER_STRING_GOES_HERE
  Q : Outport
  wires:
  Inverter1/1 -> Logical3/1
  D/1 -> Inverter1/1, Delay/1
  Delay/1 -> Logical3/2
  Logical3/1 -> Q/1

== system ofdm_txrx_supermimo_coded/OFDM Tx MIMO/Training_Data/FlexibleMod/PktBuffer_CRC1 ==
  Rst : Inport
  DataOut_Addr Vin : Inport  Port=2
  DataOut_Addr : Inport  Port=3
  Assert : xbsIndex_r4/Assert  assert_type=off  type_source=Explicitly  gui_display_data_type=Fixed-point  arith_type="Signed  (2's comp)"  n_bits=16  bin_pt=14  preci_type=Single  exp_width=8  frac_width=24  assert_rate=on  rate_source=Explicitly  period=2  output_port=on  block_type=assert
      settings: infoedit=Asserts a user-defined sample rate and/or type on a signal.<P><P>Hardware notes: In hardware this block costs nothing.  block_version=8.2.01
  Byte Selector : Subsystem
  CRC Length Calc1 : Subsystem
  CRC16 Calc : Subsystem
  CRC32 Calc : Subsystem
  Gateway Out{1..6} (6 alike) : xbsIndex_r4/Gateway Out  inherit_from_input=off  hdl_port=off  timing_constraint=None  locs_specified=off  LOCs={}  UseAsDAC=off  DACChannel='1'  block_type=gatewayout
      settings: infoedit="Gateway out block.  Converts Xilinx fixed point inputs into ouputs of type Simulink integer, double, or fixed point.<P><P>Hardware notes:  In hardware these blocks become top level output ports or are discarded, depending on how they are configured."  block_version=10.1.3
  Header Decoding1 : Subsystem
  Logical8 : xbsIndex_r4/Logical  logical_function=OR  inputs=2  en=off  latency=0  precision=Full  arith_type=Unsigned  n_bits=8  bin_pt=2  align_bp=on  dbl_ovrd=off  block_type=logical
      settings: xl_area=[0, 0, 0, 0, 0, 0, 0]  block_version=VER_STRING_GOES_HERE
  Mux : xbsIndex_r4/Mux  inputs=2  en=off  latency=0  precision=Full  arith_type="Signed  (2's comp)"  n_bits=8  bin_pt=2  quantization=Truncate  overflow=Wrap  dbl_ovrd=off  block_type=mux
      settings: xl_area=[0, 0, 0, 0, 0, 0, 0]  block_version=VER_STRING_GOES_HERE
  Packet Buffer : Subsystem
  Register{1..6} (6 alike) : xbsIndex_r4/Register  init=0  rst=off  en=off  dbl_ovrd=off  block_type=register
      settings: xl_area=[2 4 0 0 0 0 0]  block_version=VER_STRING_GOES_HERE
  Scrambling : Subsystem
  Tx Bytes : Scope  NumInputPorts=6
      settings: TimeRange=15000  YMin=0~0~-1~-5~-5~-5  YMax=3~4e+009~2~5~5~5
  NumBytes : Outport
  FullRateMasks : Outport  Port=2
  Dout : Outport  Port=3
  Info_data : Outport  Port=4
  wires:
  Header Decoding1/1(numBytes) -> CRC Length Calc1/1(NumBytes), Gateway Out5/1, NumBytes/1
  Header Decoding1/2(modMask) -> FullRateMasks/1, Gateway Out6/1
  DataOut_Addr/1 -> Packet Buffer/1(TxByteAddr), Register2/1, Register4/1
  Scrambling/1(Dout) -> Dout/1
  Mux/1 -> Scrambling/1(Din), Assert/1, Gateway Out4/1
  Packet Buffer/1(ByteOut) -> Mux/2, Header Decoding1/2(TxByte)
  DataOut_Addr Vin/1 -> Register3/1, Gateway Out2/1
  Rst/1 -> Register1/1, Gateway Out3/1
  CRC Length Calc1/3(CRC_ByteSel) -> Byte Selector/2(ByteSel)
  Byte Selector/1(1 Byte Out) -> Mux/3
  CRC Length Calc1/4(CRC32_Capt) -> CRC32 Calc/4(Capt)
  Assert/1 -> CRC16 Calc/1(ByteIn), CRC32 Calc/1(ByteIn), Info_data/1
  Register3/1 -> Header Decoding1/3(TxByteAddrVin), CRC Length Calc1/3(Vin), CRC32 Calc/2(Vin), CRC16 Calc/2(Vin)
  Register1/1 -> CRC32 Calc/3(Rst), Header Decoding1/4(Rst), CRC16 Calc/3(Rst)
  Register2/1 -> Header Decoding1/1(TxByteAddr), Register6/1, Scrambling/2(Addr)
  CRC32 Calc/1(32-bit CRC Capt) -> Byte Selector/3(CRC32)
  CRC Length Calc1/5(CRC16_Capt) -> CRC16 Calc/4(Capt)
  CRC Length Calc1/1(CRC32_En) -> Logical8/1, Byte Selector/1(CRC32_en)
  CRC Length Calc1/2(CRC16_En) -> Logical8/2
  Logical8/1 -> Mux/1
  CRC16 Calc/1(16-bit CRC Capt) -> Byte Selector/4(CRC16)
  Gateway Out4/1 -> Tx Bytes/1  "data"
  Gateway Out1/1 -> Tx Bytes/2  "addr"
  Register4/1 -> Register5/1
  Register5/1 -> Gateway Out1/1
  Register6/1 -> CRC Length Calc1/2(ByteAddr)
  Gateway Out2/1 -> Tx Bytes/3  "vin"
  Gateway Out3/1 -> Tx Bytes/4  "rst"
  Gateway Out5/1 -> Tx Bytes/5  "numBytes"
  Gateway Out6/1 -> Tx Bytes/6  "Rate"
